simulink model "ofdm_txrx_supermimo"  (Simulink 7.1)
settings: Solver=VariableStepDiscrete  SolverMode=SingleTasking  StartTime=0.0  StopTime=simOnly_simLength  RelTol=1e-3
PostLoadFcn:
  | ofdm_rx_supermimo_init
InitFcn:
  | ofdm_rx_supermimo_init
StartFcn:
  | ofdm_rx_supermimo_init
values from neighbouring files (symbols this model uses):
  Action0_Config = 0* 31*2^18 + 0*2^24 + PHY_AUTORESPONSE_REQ_GOODHDR + PHY_AUTORESPONSE_REQ_GOODPKT + PHY_AUTORESPONSE_REQ_FLAGA    [ofdm_tx_supermimo_init.m]
  Action1_Config = 0* 62*2^18 + PHY_AUTORESPONSE_REQ_GOODHDR + PHY_AUTORESPONSE_REQ_GOODPKT    [ofdm_tx_supermimo_init.m]
  Action2_Config = 0    [ofdm_tx_supermimo_init.m]
  Action3_Config = 0    [ofdm_tx_supermimo_init.m]
  Action4_Config = 0    [ofdm_tx_supermimo_init.m]
  Action5_Config = 0    [ofdm_tx_supermimo_init.m]
  ActionID_AFTransmit = 31    [ofdm_tx_supermimo_init.m]
  ActionID_Disabled = 0    [ofdm_tx_supermimo_init.m]
  ActionID_SetFlagA = 62    [ofdm_tx_supermimo_init.m]
  ActionID_SetFlagB = 61    [ofdm_tx_supermimo_init.m]
  BER_RAM_init_size = 2048    [ofdm_tx_supermimo_init.m]
  CPLength = 16    [ofdm_tx_supermimo_init.m]
  longCorr_coef_i = [-4*real(LTS) 0]    [ofdm_rx_supermimo_init.m]
  longCorr_coef_q = [4*imag(LTS) 0]    [ofdm_rx_supermimo_init.m]
  Match0_Config = 0    [ofdm_tx_supermimo_init.m]
  Match1_Config = 0    [ofdm_tx_supermimo_init.m]
  Match2_Config = 0    [ofdm_tx_supermimo_init.m]
  Match3_Config = 0    [ofdm_tx_supermimo_init.m]
  Match4_Config = 0    [ofdm_tx_supermimo_init.m]
  Match5_Config = 0    [ofdm_tx_supermimo_init.m]
  Match6_Config = 0    [ofdm_tx_supermimo_init.m]
  Match7_Config = 0    [ofdm_tx_supermimo_init.m]
  max_CP_length = 16    [ofdm_tx_supermimo_init.m]
  max_num_baseRateSymbols = 31    [ofdm_tx_supermimo_init.m]
  max_num_subcarriers = 64    [ofdm_tx_supermimo_init.m]
  max_num_trainingSymbols = 15    [ofdm_tx_supermimo_init.m]
  max_numBytes = 16384    [ofdm_tx_supermimo_init.m]
  max_OFDM_symbols = 511    [ofdm_tx_supermimo_init.m]
  modConstellation_qam16 = .75*[1 3 -1 -3]./3    [ofdm_tx_supermimo_init.m]
  modConstellation_qam64 = 0.875*[3 1 5 7 -3 -1 -5 -7]./7    [ofdm_tx_supermimo_init.m]
  modConstellation_qpsk = [1 -1]./sqrt(2)    [ofdm_tx_supermimo_init.m]
  numBaseRateSymbols = ceil(numHeaderBytes / numBytes_BaseRateOFDMSymbol)    [ofdm_tx_supermimo_init.m]
  numSubcarriers = 64    [ofdm_tx_supermimo_init.m]
  numTrainingSymbols = 2    [ofdm_tx_supermimo_init.m]
  pilot_indicies = 7 + ( (64-21) * 2^8) + ( (64-7) * 2^16) + (21 * 2^24)    (= 356068103)    [ofdm_tx_supermimo_init.m]
  pilotValues = hex2dec('A57D') + (2^16 * hex2dec('5A82'))    [ofdm_tx_supermimo_init.m]
  pkt_crossCorr_thresh = 0.7    [ofdm_rx_supermimo_init.m]
  pkt_energy_thresh = 0    [ofdm_rx_supermimo_init.m]
  pktByteNums = numHeaderBytes +  				byteIndex_numPayloadBytes(1)*2^8 +  				byteIndex_numPayloadBytes(2)*2^16 +  				byteIndex_simpleDynModMasks * 2^24    [ofdm_rx_supermimo_init.m]
  PktDet_Delay = 58+32 +      Symbol_Timing_Offset*2^7 +      0*2^16 +      (117* 2^24)    (= 1962935642)    [ofdm_rx_supermimo_init.m]
  pktTiming_count_payloadStart = pktTiming_count_lsStart + pktTiming_ls_num*pktTiming_ls_length    [ofdm_rx_supermimo_init.m]
  preamble = preamble_scale*[shortsyms_10 longsyms_2]    [ofdm_tx_supermimo_init.m]
  preamble_ROM = preamble_I_ROM + preamble_Q_ROM*2^16    [ofdm_tx_supermimo_init.m]
  RAM_init_size = max_numBytes    (= 16384)    [ofdm_tx_supermimo_init.m]
  reg_AF_Tx_Blanking = (320+80) + ( (320+80+79)*2^16)    (= 31392144)    [ofdm_rx_supermimo_init.m]
  reg_AF_Tx_Scaling = 2944    [ofdm_rx_supermimo_init.m]
  reg_Interrupt_PktBuf_Control = reg_InterruptControl +  	1 * 2^16 +  	0 * 2^24    (= 65656)    [ofdm_rx_supermimo_init.m]
  reg_PilotCalcParams = (4 * 2^16) + (minPilotChanMag)    (= 262184.96)    [ofdm_rx_supermimo_init.m]
  regRx_chanEst_minMag = ((0.5)*2^15) + ((0.5 * 2^15)*2^16)    (= 1073758208)    [ofdm_rx_supermimo_init.m]
  regRx_coarseCalcCorrection = round((1.3e-3 - 1.2982e-3)*2^32)    [ofdm_rx_supermimo_init.m]
  regRx_pilotCalcCorrection = round(1.00115 * 2^31)    [ofdm_rx_supermimo_init.m]
  regRx_preCFOoptions = 0 * 2^0 +  	1 * 2^1 +  	0    (= 2)    [ofdm_rx_supermimo_init.m]
  rx_controlBits = 1 * 2  		 + 1 * 4  		 + 0 * 8  		 + 1 * 16 * rx_SISO_Mode  		 + 1 * 32  		 + 1 * 64  		 + 1 * 128  		 + 1 * 256  		 + 1 * 512  		 + 1 * 1024  		 + 1 * 4096  		 + 0 * 16384  		 + 0 * 32768  		 + 1 * 65536  		 + 0 * 131072  		 + 0 * 262144  		 + 1 * 524288  		 + 1 * 1048576 * tx_Alamouti_Mode  		 + 0 * 2097152           + 1 * 33554432  		 + 0 * 4194304  		 + 0 * 8388608  		 + 0 * 16777216  		 + 1 *  ...(+49 chars)    [ofdm_rx_supermimo_init.m]
  Rx_PktDet_LongCorr_Params = LongCorrCounterLoad + 0*2^8 + 0*2^16 + 255*2^24    (= 4278190325)    [ofdm_rx_supermimo_init.m]
  Rx_PktDet_LongCorrThresholds = 8000    [ofdm_rx_supermimo_init.m]
  rx_postEq_scaling = round(rxScaling*2^11) * (1 + 2^16)    [ofdm_rx_supermimo_init.m]
  RxDataScrambling_Seq = [40 198 78 63 82 173 102 245 48 111 172 115 147 230 216 93 72 65 62 2 205 242 122 90 128 83 105 97 73 10 5 252]    [ofdm_tx_supermimo_init.m]
  RxReg_FixedPktLen = 0    [ofdm_rx_supermimo_init.m]
  shortSymbol_time = shortSymbol_time(1:16).'    [ofdm_tx_supermimo_init.m]
  simOnly_numSamples = length(preamble)+( (numSubcarriers+CPLength)*(numTrainingSymbols + numBaseRateSymbols + numFullRateSymbols/2) )    [ofdm_tx_supermimo_init.m]
  train = [train train]    [ofdm_tx_supermimo_init.m]
  tx_controlBits = (antB_preambleShift * 2^4) + (2^1 * tx_Alamouti_Mode) +  	(2^0 * tx_SISO_Mode) + 4*0 + 8*1 + 0*256 + 512*0 + 1024*0 +  	0*hex2dec('1000') + 0*hex2dec('2000') + 0*hex2dec('4000') +  	1*hex2dec('8000') + 1*hex2dec('10000') + hex2dec('20000') +  	16*hex2dec('100000')    [ofdm_tx_supermimo_init.m]
  tx_delays = (50*2^0) + (0*2^8) + (30*2^12)    (= 122930)    [ofdm_tx_supermimo_init.m]
  tx_scaling = (3072) + (12288 * 2^16)    (= 805309440)    [ofdm_tx_supermimo_init.m]
  TxDataScrambling_Seq = [40 198 78 63 82 173 102 245 48 111 172 115 147 230 216 93 72 65 62 2 205 242 122 90 128 83 105 97 73 10 5 252]    [ofdm_tx_supermimo_init.m]
  txfilt_firpm = 0.95*txfilt_firpm./(max(txfilt_firpm))    [ofdm_tx_supermimo_init.m]
  txfilt_intfilt = [0 intfilt(4,4,.8)]    [ofdm_tx_supermimo_init.m]
  txReg_symbolCounts = (2^8 *numBaseRateSymbols ) + numTrainingSymbols    [ofdm_tx_supermimo_init.m]
  TxRx_FFTScaling = (3 * 2^0) +  	(2 * 2^2) +  	(1 * 2^4) +  	(1 * 2^6) +  	(1 * 2^8) +  	(0 * 2^10)    (= 347)    [ofdm_tx_supermimo_init.m]
undefined symbols (not defined in the file or in its folder): AntA, AntB, BaseRate, Down, gcbh, numBuffs, numBytes, Up, xlBlockLoadCallback, xlGetSimulinkPeriod, xlipgetpartsetting
libraries: xbsIndex_r4 x6035, simulink x4, dspsigops x4, commchan3 x2, dspsrcs4 x2, commrflib2 x2
(part 1 of 61)
settings Model: Creator=murphpo  LastModifiedBy=Administrator
settings DataIOCC: LimitDataPoints=off  SaveOutput=off  SignalLogging=off  SaveTime=off
settings OptimizationCC: InlineParams=on
settings DebuggingCC: SaveWithParameterizedLinksMsg=none

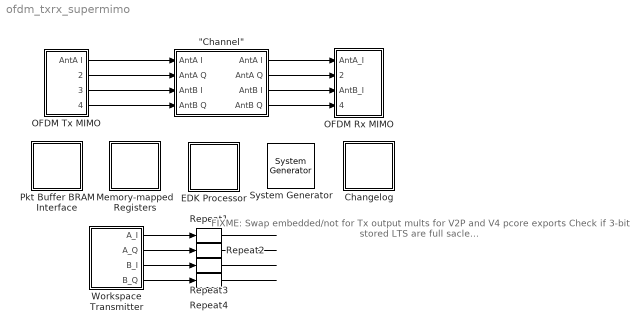
== system ofdm_txrx_supermimo ==
  note: FIXME: Swap embedded/not
      | for Tx output mults for V2P and V4
      | pcore exports
      |
      | Check if 3-bit stored LTS are full sacle...
  " System Generator" : xbsIndex_r4/ System Generator  Tag=genX  SystemSampleTime=-1  xilinxfamily=virtex2p  part=xc2vp70  speed=-6  package=ff1517  synthesis_tool=XST  clock_wrapper=Clock Enables  directory=./OFDM_txrx_v15_v50  testbench=off  simulink_period=1  sysclk_period=10  dcm_input_clock_period=100  incr_netlist=off  trim_vbits=Everywhere in SubSystem  dbl_ovrd=According to Block Masks  core_generation=According to Block Masks  run_coregen=off  deprecated_control=off  eval_field=0  block_type=sysgen
      settings: block_version=10.1.3
  "\"Channel\"" : Subsystem  mask{h=[1 0; 1 0] * 0.45 %[1 0; 0 0] * 0.5 %, freqOffsetA=0, freqOffsetB=0, awgnSNR=500, phNoise=-5000}
      mask prompts: h "2x2 Channel Matrix"; freqOffsetA "Freq Offset A (phase inc [0,1])"; freqOffsetB "Freq Offset B (phase inc [0,1])"; awgnSNR "SNR (dB)"; phNoise "Phase Noise (dBc @ 100Hz)"
      mask help: This block implements a simple static 2x2 channel. The one parameter sets the 2x2 channel matrix. h=[1 0;0 1] would be perfectly orthogonal channels. The norm of the matrix should be 1 to avoid overflow.
  Changelog : Subsystem
  EDK Processor : Subsystem  LoadFcn=xlBlockLoadCallback(gcbh);  mask(Xilinx EDK Processor Block){mode=EDK pcore generation, xmp=, MemVisToProc=<qt bgcolor="#FFFFFF"><div><img src="C:\Xilinx\10.1\DSP_Tools\common\bin\..\..\sysgen\data\images\registerplus.gif"> &lt;&lt;Rx_AF_Blanking_w&gt;&gt;<br></div><div><img src="C:\Xilinx\10.1\DSP_Tools\common\bin\..\..\sysgen\data\images\registerplus.gif"> &lt;&lt;Rx_AF_TxScaling_w&gt;&gt;<br></div><div><img src="C:\Xilinx\10.1\DSP_Tools\common\bin\..\..\sysgen\data\images\registerplus.gif"> &lt;&lt;Rx_BER_Errors&gt;&gt;<br></div><div><img src="C:\Xilinx\10.1\DSP_Tools\common\bin\..\..\sysgen\data\images\registerplus.gif"> &lt;&lt;Rx_BER_TotalBits&gt;&gt;<br></div><div><img src="C:\Xilinx\10.1\DSP_Tools\common\bin\..\..\sysgen\data\images\registerplus.gif"> &lt;&lt;Rx_ChanEst_MinMag_w&gt;&gt;<br></div><div><img src="C:\Xilinx\10.1\DSP_Tools\common\bin\..\..\sysgen\data\images\registerplus.gif"> &lt;&lt;Rx_Constellation_Scaling_w&gt;&gt;<br></div><div><img src="C:\Xilinx\10.1\DSP_Tools\common\bin\..\..\sysgen\data\images\registerplus.gif"> &lt;&lt;Rx_ControlBits_r&gt;&gt;<br></div><div><img src="C:\Xilinx\10.1\DSP_Tools\common\bin\..\..\sysgen\data\images\registerplus.gif"> &lt;&lt;Rx_ControlBits_w&gt;&gt;<br></div><div><img src="C:\Xilinx\10.1\DSP_Tools\common\bin\..\..\sysgen\data\images\registerplus.gif"> &lt;&lt;Rx_FixedPktLen_w&gt;&gt;<br></div><div><img src="C:\Xilinx\10.1\DSP_Tools\common\bin\..\..\sysgen\data\images\registerplus.gif"> &lt;&lt;Rx_Gains&gt;&gt;<br></div><div><img src="C:\Xilinx\10.1\DSP_Tools\common\bin\..\..\sysgen\data\images\registerplus.gif"> &lt;&lt;Rx_OFDM_SymbolCounts_r&gt;&gt;<br></div><div><img src="C:\Xilinx\10.1\DSP_Tools\common\bin\..\..\sysgen\data\images\registerplus.gif"> &lt;&lt;Rx_OFDM_SymbolCounts_w&gt;&gt;<br></div><div><img src="C:\Xilinx\10.1\DSP_Tools\common\bin\..\..\sysgen\data\images\registerplus.gif"> &lt;&lt;Rx_PilotCalcParams_w&gt;&gt;<br></div><div><img src="C:\Xilinx\10.1\DSP_Tools\common\bin\..\..\sysgen\data\images\registerplus.gif"> &lt;&lt;Rx_PktDet_Delay_r&gt;&gt;<br></div><div><img src="C:\Xilinx\10.1\DSP_Tools\common\bin\..\..\sysgen\data\images\registerplus.gif"> &lt;&lt;Rx_PktDet_Delay_w&gt;&gt;<br></div><div><img src="C:\Xilinx\10.1\DSP_Tools\common\bin\..\..\sysgen\data\images\registerplus.gif"> &lt;&lt;Rx_PktDet_LongCorr_Params_r&gt;&gt;<br></div><div><img src="C:\Xilinx\10.1\DSP_Tools\common\bin\..\..\sysgen\data\images\registerplus.gif"> &lt;&lt;Rx_PktDet_LongCorr_Params_w&gt;&gt;<br></div><div><img src="C:\Xilinx\10.1\DSP_Tools\common\bin\..\..\sysgen\data\images\registerplus.gif"> &lt;&lt;Rx_PktDet_LongCorr_Thresholds_w&gt;&gt;<br></div><div><img src="C:\Xilinx\10.1\DSP_Tools\common\bin\..\..\sysgen\data\images\registerplus.gif"> &lt;&lt;Rx_PreCFO_Options_w&gt;&gt;<br></div><div><img src="C:\Xilinx\10.1\DSP_Tools\common\bin\..\..\sysgen\data\images\registerplus.gif"> &lt;&lt;Rx_PreCFO_PilotCalcCorrection_w&gt;&gt;<br></div><div><img src="C:\Xilinx\10.1\DSP_Tools\common\bin\..\..\sysgen\data\images\registerplus.gif"> &lt;&lt;Rx_coarseCFO_correction_w&gt;&gt;<br></div><div><img src="C:\Xilinx\10.1\DSP_Tools\common\bin\..\..\sysgen\data\images\registerplus.gif"> &lt;&lt;Rx_coarseCFOest&gt;&gt;<br></div><div><img src="C:\Xilinx\10.1\DSP_Tools\common\bin\..\..\sysgen\data\images\registerplus.gif"> &lt;&lt;Rx_pilotCFOest&gt;&gt;<br></div><div><img src="C:\Xilinx\10.1\DSP_Tools\common\bin\..\..\sysgen\data\images\registerplus.gif"> &lt;&lt;Rx_pktByteNums_r&gt;&gt;<br></div><div><img src="C:\Xilinx\10.1\DSP_Tools\common\bin\..\..\sysgen\data\images\registerplus.gif"> &lt;&lt;Rx_pktByteNums_w&gt;&gt;<br></div><div><img src="C:\Xilinx\10.1\DSP_Tools\common\bin\..\..\sysgen\data\images\registerplus.gif"> &lt;&lt;Rx_pktDetEventCount&gt;&gt;<br></div><div><img src="C:\Xilinx\10.1\DSP_Tools\common\bin\..\..\sysgen\data\images\registerplus.gif"> &lt;&lt;Rx_pktDet_Tresholds_w&gt;&gt;<br></div><div><img src="C:\Xilinx\10.1\DSP_Tools\common\bin\..\..\sysgen\data\images\registerplus.gif"> &lt;&lt;Rx_pktDone_interruptStatus&gt;&gt;<br></div><div><img src="C:\Xilinx\10.1\DSP_Tools\common\bin\..\..\sysgen\data\images\registerplus.gif"> &lt;&lt;TxRx_AutoReply_Action0_r&gt;&gt;<br></div><div><img src="C:\Xilinx\10.1\DSP_Tools\common\bin\..\..\sysgen\data\images\registerplus.gif"> &lt;&lt;TxRx_AutoReply_Action0_w&gt;&gt;<br></div><div><img src="C:\Xilinx\10.1\DSP_Tools\common\bin\..\..\sysgen\data\images\registerplus.gif"> &lt;&lt;TxRx_AutoReply_Action1_r&gt;&gt;<br></div><div><img src="C:\Xilinx\10.1\DSP_Tools\common\bin\..\..\sysgen\data\images\registerplus.gif"> &lt;&lt;TxRx_AutoReply_Action1_w&gt;&gt;<br></div><div><img src="C:\Xilinx\10.1\DSP_Tools\common\bin\..\..\sysgen\data\images\registerplus.gif"> &lt;&lt;TxRx_AutoReply_Action2_r&gt;&gt;<br></div><div><img src="C:\Xilinx\10.1\DSP_Tools\common\bin\..\..\sysgen\data\images\registerplus.gif"> &lt;&lt;TxRx_AutoReply_Action2_w&gt;&gt;<br></div><div><img src="C:\Xilinx\10.1\DSP_Tools\common\bin\..\..\sysgen\data\images\registerplus.gif"> &lt;&lt;TxRx_AutoReply_Action3_r&gt;&gt;<br></div><div><img src="C:\Xilinx\10.1\DSP_Tools\common\bin\..\..\sysgen\data\images\registerplus.gif"> &lt;&lt;TxRx_AutoReply_Action3_w&gt;&gt;<br></div><div><img src="C:\Xilinx\10.1\DSP_Tools\common\bin\..\..\sysgen\data\images\registerplus.gif"> &lt;&lt;TxRx_AutoReply_Action4_r&gt;&gt;<br></div><div><img src="C:\Xilinx\10.1\DSP_Tools\common\bin\..\..\sysgen\data\images\registerplus.gif"> &lt;&lt;TxRx_AutoReply_Action4_w&gt;&gt;<br></div><div><img src="C:\Xilinx\10.1\DSP_Tools\common\bin\..\..\sysgen\data\images\registerplus.gif"> &lt;&lt;TxRx_AutoReply_Action5_r&gt;&gt;<br></div><div><img src="C:\Xilinx\10.1\DSP_Tools\common\bin\..\..\sysgen\data\images\registerplus.gif"> &lt;&lt;TxRx_AutoReply_Action5_w&gt;&gt;<br></div><div><img src="C:\Xilinx\10.1\DSP_Tools\common\bin\..\..\sysgen\data\images\registerplus.gif"> &lt;&lt;TxRx_AutoReply_Match0_r&gt;&gt;<br></div><div><img src="C:\Xilinx\10.1\DSP_Tools\common\bin\..\..\sysgen\data\images\registerplus.gif"> &lt;&lt;TxRx_AutoReply_Match0_w&gt;&gt;<br></div><div><img src="C:\Xilinx\10.1\DSP_Tools\common\bin\..\..\sysgen\data\images\registerplus.gif"> &lt;&lt;TxRx_AutoReply_Match1_r&gt;&gt;<br></div><div><img src="C:\Xilinx\10.1\DSP_Tools\common\bin\..\..\sysgen\data\images\registerplus.gif"> &lt;&lt;TxRx_AutoReply_Match1_w&gt;&gt;<br></div><div><img src="C:\Xilinx\10.1\DSP_Tools\common\bin\..\..\sysgen\data\images\registerplus.gif"> &lt;&lt;TxRx_AutoReply_Match2_r&gt;&gt;<br></div><div><img src="C:\Xilinx\10.1\DSP_Tools\common\bin\..\..\sysgen\data\images\registerplus.gif"> &lt;&lt;TxRx_AutoReply_Match2_w&gt;&gt;<br></div><div><img src="C:\Xilinx\10.1\DSP_Tools\common\bin\..\..\sysgen\data\images\registerplus.gif"> &lt;&lt;TxRx_AutoReply_Match3_r&gt;&gt;<br></div><div><img src="C:\Xilinx\10.1\DSP_Tools\common\bin\..\..\sysgen\data\images\registerplus.gif"> &lt;&lt;TxRx_AutoReply_Match3_w&gt;&gt;<br></div><div><img src="C:\Xilinx\10.1\DSP_Tools\common\bin\..\..\sysgen\data\images\registerplus.gif"> &lt;&lt;TxRx_AutoReply_Match4_r&gt;&gt;<br></div><div><img src="C:\Xilinx\10.1\DSP_Tools\common\bin\..\..\sysgen\data\images\registerplus.gif"> &lt;&lt;TxRx_AutoReply_Match4_w&gt;&gt;<br></div><div><img src="C:\Xilinx\10.1\DSP_Tools\common\bin\..\..\sysgen\data\images\registerplus.gif"> &lt;&lt;TxRx_AutoReply_Match5_r&gt;&gt;<br></div><div><img src="C:\Xilinx\10.1\DSP_Tools\common\bin\..\..\sysgen\data\images\registerplus.gif"> &lt;&lt;TxRx_AutoReply_Match5_w&gt;&gt;<br></div><div><img src="C:\Xilinx\10.1\DSP_Tools\common\bin\..\..\sysgen\data\images\registerplus.gif"> &lt;&lt;TxRx_AutoReply_Match6_r&gt;&gt;<br></div><div><img src="C:\Xilinx\10.1\DSP_Tools\common\bin\..\..\sysgen\data\images\registerplus.gif"> &lt;&lt;TxRx_AutoReply_Match6_w&gt;&gt;<br></div><div><img src="C:\Xilinx\10.1\DSP_Tools\common\bin\..\..\sysgen\data\images\registerplus.gif"> &lt;&lt;TxRx_AutoReply_Match7_r&gt;&gt;<br></div><div><img src="C:\Xilinx\10.1\DSP_Tools\common\bin\..\..\sysgen\data\images\registerplus.gif"> &lt;&lt;TxRx_AutoReply_Match7_w&gt;&gt;<br></div><div><img src="C:\Xilinx\10.1\DSP_Tools\common\bin\..\..\sysgen\data\images\registerplus.gif"> &lt;&lt;TxRx_FFT_Scaling_w&gt;&gt;<br></div><div><img src="C:\Xilinx\10.1\DSP_Tools\common\bin\..\..\sysgen\data\images\registerplus.gif"> &lt;&lt;TxRx_Interrupt_PktBuf_Ctrl_r&gt;&gt;<br></div><div><img src="C:\Xilinx\10.1\DSP_Tools\common\bin\..\..\sysgen\data\images\registerplus.gif"> &lt;&lt;TxRx_Interrupt_PktBuf_Ctrl_w&gt;&gt;<br></div><div><img src="C:\Xilinx\10.1\DSP_Tools\common\bin\..\..\sysgen\data\images\registerplus.gif"> &lt;&lt;TxRx_Pilots_Index_w&gt;&gt;<br></div><div><img src="C:\Xilinx\10.1\DSP_Tools\common\bin\..\..\sysgen\data\images\registerplus.gif"> &lt;&lt;TxRx_Pilots_Values_w&gt;&gt;<br></div><div><img src="C:\Xilinx\10.1\DSP_Tools\common\bin\..\..\sysgen\data\images\registerplus.gif"> &lt;&lt;Tx_ControlBits_r&gt;&gt;<br></div><div><img src="C:\Xilinx\10.1\DSP_Tools\common\bin\..\..\sysgen\data\images\registerplus.gif"> &lt;&lt;Tx_ControlBits_w&gt;&gt;<br></div><div><img src="C:\Xilinx\10.1\DSP_Tools\common\bin\..\..\sysgen\data\images\registerplus.gif"> &lt;&lt;Tx_Delays_r&gt;&gt;<br></div><div><img src="C:\Xilinx\10.1\DSP_Tools\common\bin\..\..\sysgen\data\images\registerplus.gif"> &lt;&lt;Tx_Delays_w&gt;&gt;<br></div><div><img src="C:\Xilinx\10.1\DSP_Tools\common\bin\..\..\sysgen\data\images\registerplus.gif"> &lt;&lt;Tx_OFDM_SymCounts_r&gt;&gt;<br></div><div><img src="C:\Xilinx\10.1\DSP_Tools\common\bin\..\..\sysgen\data\images\registerplus.gif"> &lt;&lt;Tx_OFDM_SymCounts_w&gt;&gt;<br></div><div><img src="C:\Xilinx\10.1\DSP_Tools\common\bin\..\..\sysgen\data\images\registerplus.gif"> &lt;&lt;Tx_PktRunning&gt;&gt;<br></div><div><img src="C:\Xilinx\10.1\DSP_Tools\common\bin\..\..\sysgen\data\images\registerplus.gif"> &lt;&lt;Tx_Scaling_w&gt;&gt;<br></div><div><img src="C:\Xilinx\10.1\DSP_Tools\common\bin\..\..\sysgen\data\images\registerplus.gif"> &lt;&lt;Tx_Start_Reset_Control_r&gt;&gt;<br></div><div><img src="C:\Xilinx\10.1\DSP_Tools\common\bin\..\..\sysgen\data\images\registerplus.gif"> &lt;&lt;Tx_Start_Reset_Control_w&gt;&gt;<br></div><div><img src="C:\Xilinx\10.1\DSP_Tools\common\bin\..\..\sysgen\data\images\registerplus.gif"> &lt;&lt;midPacketRSSI&gt;&gt;<br></div><div><img src="C:\Xilinx\10.1\DSP_Tools\common\bin\..\..\sysgen\data\images\registerplus.gif"> &lt;&lt;pktDet_controlBits_r&gt;&gt;<br></div><div><img src="C:\Xilinx\10.1\DSP_Tools\common\bin\..\..\sysgen\data\images\registerplus.gif"> &lt;&lt;pktDet_controlBits_w&gt;&gt;<br></div><div><img src="C:\Xilinx\10.1\DSP_Tools\common\bin\..\..\sysgen\data\images\registerplus.gif"> &lt;&lt;pktDet_durations_r&gt;&gt;<br></div><div><img src="C:\Xilinx\10.1\DSP_Tools\common\bin\..\..\sysgen\data\images\registerplus.gif"> &lt;&lt;pktDet_durations_w&gt;&gt;<br></div><div><img src="C:\Xilinx\10.1\DSP_Tools\common\bin\..\..\sysgen\data\images\registerplus.gif"> &lt;&lt;pktDet_status&gt;&gt;<br></div><div><img src="C:\Xilinx\10.1\DSP_Tools\common\bin\..\..\sysgen\data\images\registerplus.gif"> &lt;&lt;pktDet_thresholds_r&gt;&gt;<br></div><div><img src="C:\Xilinx\10.1\DSP_Tools\common\bin\..\..\sysgen\data\images\registerplus.gif"> &lt;&lt;pktDet_thresholds_w&gt;&gt;<br></div><div><img src="C:\Xilinx\10.1\DSP_Tools\common\bin\..\..\sysgen\data\images\memoryplus.gif"> &lt;&lt;ChannelEstimates&gt;&gt;<br></div><div><img src="C:\Xilinx\10.1\DSP_Tools\common\bin\..\..\sysgen\data\images\memoryplus.gif"> &lt;&lt;EVM_perSC&gt;&gt;<br></div><div><img src="C:\Xilinx\10.1\DSP_Tools\common\bin\..\..\sysgen\data\images\memoryplus.gif"> &lt;&lt;EVM_perSym&gt;&gt;<br></div><div><img src="C:\Xilinx\10.1\DSP_Tools\common\bin\..\..\sysgen\data\images\memoryplus.gif"> &lt;&lt;PktBufFreqOffsets&gt;&gt;<br></div><div><img src="C:\Xilinx\10.1\DSP_Tools\common\bin\..\..\sysgen\data\images\memoryplus.gif"> &lt;&lt;RxModulation&gt;&gt;<br></div><div><img src="C:\Xilinx\10.1\DSP_Tools\common\bin\..\..\sysgen\data\images\memoryplus.gif"> &lt;&lt;TxHeaderTranslate&gt;&gt;<br></div><div><img src="C:\Xilinx\10.1\DSP_Tools\common\bin\..\..\sysgen\data\images\memoryplus.gif"> &lt;&lt;TxModulation&gt;&gt;<br></div></qt>, AvailableMemories=<empty>, portInterfaceTable={'exposed'=>[],'portdir'=>[],'portname'=>[],'shortname'=>[]}, bus_type_sgadvanced=, bus_type=PLB, baseaddr=0x80000000, baseaddr_lock_sgadvanced=, baseaddr_lock=off, dual_clock_sgadvanced=, dual_clock=off, reg_readback_sgadvanced=, reg_readback=off, ucf_file=, inheritDeviceType_sgadvanced=, inheritDeviceType=off, clock_name=plb, internalPortList={}, resetPolarity=0, memxtable=|, procinfo={'xmliface'=>'Xilinx//microblaze//iface.xml'}, memmapdirty=off, blockname=, xpsintstyle=default, has_advanced_control=0, sggui_pos=20,20,383,441, block_type=edkprocessor, block_version=2.5, sg_icon_stat=47,45,-1,-1,white,blue,0,07734,right, sg_mask_display=|, sg_list_contents={'table'=>{'AvailableMemories'=>'popup(<empty>)','userSelections'=>{'AvailableMemories'=>'<empty>'}}}, sg_blockgui_xml=}
      mask memxtable=|
      | {'mlist'=>['ofdm_txrx_supermimo/Memory-mapped
      | Registers/Rx Registers/Write-Only Register4/From Register','ofdm_txrx_supermimo/Memory-mapped
      | Registers/Rx Registers/Write-Only Register6/From Register','ofdm_txrx_supermimo/Memory-mapped
      | Registers/Rx Registers/Read Only Register1/To Register','ofdm_txrx_supermimo/Memory-mapped
      | Registers/Rx Registers/Read Only Register2/To Register','ofdm_txrx_supermimo/Memory-mapped
      | Registers/Rx Registers/Write-Only Register8/From Register','ofdm_txrx_supermimo/Memory-mapped
      | Registers/Rx Registers/Write-Only Register1/From Register','ofdm_txrx_supermimo/Memory-mapped
      | Registers/Rx Registers/Read-Write Register/To Register','ofdm_txrx_supermimo/Memory-mapped
      | Registers/Rx Registers/Read-Write Register/From Register','ofdm_txrx_supermimo/Memory-mapped
      | Registers/Rx Registers/Write-Only Register10/From Register','ofdm_txrx_supermimo/Memory-mapped
      | Registers/Rx Registers/Read Only Register/To Register','ofdm_txrx_supermimo/Memory-mapped
      | Registers/TxRx Registers/Read-Write Register4/To Register','ofdm_txrx_supermimo/Memory-mapped
      | Registers/TxRx Registers/Read-Write Register4/From Register','ofdm_txrx_supermimo/Memory-mapped
      | Registers/Rx Registers/Write-Only Register5/From Register','ofdm_txrx_supermimo/Memory-mapped
      | Registers/Rx Registers/Read-Write Register3/To Register','ofdm_txrx_supermimo/Memory-mapped
      | Registers/Rx Registers/Read-Write Register3/From Register','ofdm_txrx_supermimo/Memory-mapped
      | Registers/Rx Registers/Read-Write Register1/To Register','ofdm_txrx_supermimo/Memory-mapped
      | Registers/Rx Registers/Read-Write Register1/From Register','ofdm_txrx_supermimo/Memory-mapped
      | Registers/Rx Registers/Write-Only Register9/From Register','ofdm_txrx_supermimo/Memory-mapped
      | Registers/Rx Registers/Write-Only Register7/From Register','ofdm_txrx_supermimo/Memory-mapped
      | Registers/Rx Registers/Write-Only Register2/From Register','ofdm_txrx_supermimo/Memory-mapped
      | Registers/Rx Registers/Write-Only Register3/From Register','ofdm_txrx_supermimo/Memory-mapped
      | Registers/Rx Registers/Read Only Register5/To Register','ofdm_txrx_supermimo/Memory-mapped
      | Registers/Rx Registers/Read Only Register4/To Register','ofdm_txrx_supermimo/Memory-mapped
      | Registers/Rx Registers/Read-Write Register2/To Register','ofdm_txrx_supermimo/Memory-mapped
      | Registers/Rx Registers/Read-Write Register2/From Register','ofdm_txrx_supermimo/Memory-mapped
      | Registers/Rx Registers/Read Only Register6/To Register','ofdm_txrx_supermimo/Memory-mapped
      | Registers/Rx Registers/Write-Only Register/From Register','ofdm_txrx_supermimo/Memory-mapped
      | Registers/Rx Registers/Read Only Register3/To Register','ofdm_txrx_supermimo/Memory-mapped
      | Registers/AutoReply Registers/Read-Write Register7/To Register','ofdm_txrx_supermimo/Memory-mapped
      | Registers/AutoReply Registers/Read-Write Register7/From Register','ofdm_txrx_supermimo/Memory-mapped
      | Registers/AutoReply Registers/Read-Write Register8/To Register','ofdm_txrx_supermimo/Memory-mapped
      | Registers/AutoReply Registers/Read-Write Register8/From Register','ofdm_txrx_supermimo/Memory-mapped
      | Registers/AutoReply Registers/Read-Write Register9/To Register','ofdm_txrx_supermimo/Memory-mapped
      | Registers/AutoReply Registers/Read-Write Register9/From Register','ofdm_txrx_supermimo/Memory-mapped
      | Registers/AutoReply Registers/Read-Write Register10/To Register','ofdm_txrx_supermimo/Memory-mapped
      | Registers/AutoReply Registers/Read-Write Register10/From Register','ofdm_txrx_supermimo/Memory-mapped
      | Registers/AutoReply Registers/Read-Write Register12/To Register','ofdm_txrx_supermimo/Memory-mapped
      | Registers/AutoReply Registers/Read-Write Register12/From Register','ofdm_txrx_supermimo/Memory-mapped
      | Registers/AutoReply Registers/Read-Write Register11/To Register','ofdm_txrx_supermimo/Memory-mapped
      | Registers/AutoReply Registers/Read-Write Register11/From Register','ofdm_txrx_supermimo/Memory-mapped
      | Registers/AutoReply Registers/Read-Write Register5/To Register','ofdm_txrx_supermimo/Memory-mapped
      | Registers/AutoReply Registers/Read-Write Register5/From Register','ofdm_txrx_supermimo/Memory-mapped
      | Registers/AutoReply Registers/Read-Write Register1/To Register','ofdm_txrx_supermimo/Memory-mapped
      | Registers/AutoReply Registers/Read-Write Register1/From Register','ofdm_txrx_supermimo/Memory-mapped
      | Registers/AutoReply Registers/Read-Write Register2/To Register','ofdm_txrx_supermimo/Memory-mapped
      | Registers/AutoReply Registers/Read-Write Register2/From Register','ofdm_txrx_supermimo/Memory-mapped
      | Registers/AutoReply Registers/Read-Write Register6/To Register','ofdm_txrx_supermimo/Memory-mapped
      | Registers/AutoReply Registers/Read-Write Register6/From Register','ofdm_txrx_supermimo/Memory-mapped
      | Registers/AutoReply Registers/Read-Write Register3/To Register','ofdm_txrx_supermimo/Memory-mapped
      | Registers/AutoReply Registers/Read-Write Register3/From Register','ofdm_txrx_supermimo/Memory-mapped
      | Registers/AutoReply Registers/Read-Write Register4/To Register','ofdm_txrx_supermimo/Memory-mapped
      | Registers/AutoReply Registers/Read-Write Register4/From Register','ofdm_txrx_supermimo/Memory-mapped
      | Registers/AutoReply Registers/Read-Write Register13/To Register','ofdm_txrx_supermimo/Memory-mapped
      | Registers/AutoReply Registers/Read-Write Register13/From Register','ofdm_txrx_supermimo/Memory-mapped
      | Registers/AutoReply Registers/Read-Write Register14/To Register','ofdm_txrx_supermimo/Memory-mapped
      | Registers/AutoReply Registers/Read-Write Register14/From Register','ofdm_txrx_supermimo/Memory-mapped
      | Registers/TxRx Registers/Write-Only Register/From Register','ofdm_txrx_supermimo/Memory-mapped
      | Registers/TxRx Registers/Read-Write Register/To Register','ofdm_txrx_supermimo/Memory-mapped
      | Registers/TxRx Registers/Read-Write Register/From Register','ofdm_txrx_supermimo/Memory-mapped
      | Registers/TxRx Registers/Write-Only Register2/From Register','ofdm_txrx_supermimo/Memory-mapped
      | Registers/TxRx Registers/Write-Only Register4/From Register','ofdm_txrx_supermimo/Memory-mapped
      | Registers/Tx Registers/Read-Write Register1/To Register','ofdm_txrx_supermimo/Memory-mapped
      | Registers/Tx Registers/Read-Write Register1/From Register','ofdm_txrx_supermimo/Memory-mapped
      | Registers/Tx Registers/Read-Write Register2/To Register','ofdm_txrx_supermimo/Memory-mapped
      | Registers/Tx Registers/Read-Write Register2/From Register','ofdm_txrx_supermimo/Memory-mapped
      | Registers/TxRx Registers/Read-Write Register2/To Register','ofdm_txrx_supermimo/Memory-mapped
      | Registers/TxRx Registers/Read-Write Register2/From Register','ofdm_txrx_supermimo/Memory-mapped
      | Registers/Tx Registers/Read Only Register/To Register','ofdm_txrx_supermimo/Memory-mapped
      | Registers/Tx Registers/Write-Only Register1/From Register','ofdm_txrx_supermimo/Memory-mapped
      | Registers/Tx Registers/Read-Write Register/To Register','ofdm_txrx_supermimo/Memory-mapped
      | Registers/Tx Registers/Read-Write Register/From Register','ofdm_txrx_supermimo/OFDM Rx MIMO/Packet_Detection/RSSI-based PktDet/Mid-Packet
      | RSSI Register/To Register2','ofdm_txrx_supermimo/Memory-mapped
      | Registers/Rx Registers/Pkt Detector Registers/Read-Write Register1/To Register','ofdm_txrx_supermimo/Memory-mapped
      | Registers/Rx Registers/Pkt Detector Registers/Read-Write Register1/From Register','ofdm_txrx_supermimo/Memory-mapped
      | Registers/Rx Registers/Pkt Detector Registers/Read-Write Register2/To Register','ofdm_txrx_supermimo/Memory-mapped
      | Registers/Rx Registers/Pkt Detector Registers/Read-Write Register2/From Register','ofdm_txrx_supermimo/Memory-mapped
      | Registers/Rx Registers/Pkt Detector Registers/To Register','ofdm_txrx_supermimo/Memory-mapped
      | Registers/Rx Registers/Pkt Detector Registers/Read-Write Register5/To Register','ofdm_txrx_supermimo/Memory-mapped
      | Registers/Rx Registers/Pkt Detector Registers/Read-Write Register5/From Register','ofdm_txrx_supermimo/OFDM Rx MIMO/FFT & Chan Est/Channel Estimation
      | & Pilot Processing/Chan Est Buffer
      | & Mag Calc/Shared Memory','ofdm_txrx_supermimo/OFDM Rx MIMO/Equalizer & Packetizer/Packet_Constructor/EVM Calc/EVM per Subcarrier/Shared Memory','ofdm_txrx_supermimo/OFDM Rx MIMO/Equalizer & Packetizer/Packet_Constructor/EVM Calc/EVM per Symbol/Shared Memory','ofdm_txrx_supermimo/OFDM Rx MIMO/Coarse Freq Correction/PreSpin CFO Sel/Shared Memory','ofdm_txrx_supermimo/OFDM Rx MIMO/Equalizer & Packetizer/Packet_Constructor/Modulation RAM/Modulation Masks/Shared Memory','ofdm_txrx_supermimo/OFDM Tx MIMO/Training_Data/FlexibleMod/PktBuffer_CRC1/Packet Buffer/AutoTx
      | Header Translation/Shared Memory','ofdm_txrx_supermimo/OFDM Tx MIMO/Training_Data/FlexibleMod/control/Tx Modulation/Shared Memory for
      | Modulation Masks/Shared Memory'],'mlname'=>['\'Rx_AF_Blanking_w\'','\'Rx_AF_TxScaling_w\'','\'Rx_BER_Errors\'','\'Rx_BER_TotalBits\'','\'Rx_ChanEst_MinMag_w\'','\'Rx_Constellation_Scaling_w\'','\'Rx_ControlBits_r\'','\'Rx_ControlBits_w\'','\'Rx_FixedPktLen_w\'','\'Rx_Gains\'','\'Rx_OFDM_SymbolCounts_r\'','\'Rx_OFDM_SymbolCounts_w\'','\'Rx_PilotCalcParams_w\'','\'Rx_PktDet_Delay_r\'','\'Rx_PktDet_Delay_w\'','\'Rx_PktDet_LongCorr_Params_r\'','\'Rx_PktDet_LongCorr_Params_w\'','\'Rx_PktDet_LongCorr_Thresholds_w\'','\'Rx_PreCFO_Options_w\'','\'Rx_PreCFO_PilotCalcCorrection_w\'','\'Rx_coarseCFO_correction_w\'','\'Rx_coarseCFOest\'','\'Rx_pilotCFOest\'','\'Rx_pktByteNums_r\'','\'Rx_pktByteNums_w\'','\'Rx_pktDetEventCount\'','\'Rx_pktDet_Tresholds_w\'','\'Rx_pktDone_interruptStatus\'','\'TxRx_AutoReply_Action0_r\'','\'TxRx_AutoReply_Action0_w\'','\'TxRx_AutoReply_Action1_r\'','\'TxRx_AutoReply_Action1_w\'','\'TxRx_AutoReply_Action2_r\'','\'TxRx_AutoReply_Action2_w\'','\'TxRx_AutoReply_Action3_r\'','\'TxRx_AutoReply_Action3_w\'','\'TxRx_AutoReply_Action4_r\'','\'TxRx_AutoReply_Action4_w\'','\'TxRx_AutoReply_Action5_r\'','\'TxRx_AutoReply_Action5_w\'','\'TxRx_AutoReply_Match0_r\'','\'TxRx_AutoReply_Match0_w\'','\'TxRx_AutoReply_Match1_r\'','\'TxRx_AutoReply_Match1_w\'','\'TxRx_AutoReply_Match2_r\'','\'TxRx_AutoReply_Match2_w\'','\'TxRx_AutoReply_Match3_r\'','\'TxRx_AutoReply_Match3_w\'','\'TxRx_AutoReply_Match4_r\'','\'TxRx_AutoReply_Match4_w\'','\'TxRx_AutoReply_Match5_r\'','\'TxRx_AutoReply_Match5_w\'','\'TxRx_AutoReply_Match6_r\'','\'TxRx_AutoReply_Match6_w\'','\'TxRx_AutoReply_Match7_r\'','\'TxRx_AutoReply_Match7_w\'','\'TxRx_FFT_Scaling_w\'','\'TxRx_Interrupt_PktBuf_Ctrl_r\'','\'TxRx_Interrupt_PktBuf_Ctrl_w\'','\'TxRx_Pilots_Index_w\'','\'TxRx_Pilots_Values_w\'','\'Tx_ControlBits_r\'','\'Tx_ControlBits_w\'','\'Tx_Delays_r\'','\'Tx_Delays_w\'','\'Tx_OFDM_SymCounts_r\'','\'Tx_OFDM_SymCounts_w\'','\'Tx_PktRunning\'','\'Tx_Scaling_w\'','\'Tx_Start_Reset_Control_r\'','\'Tx_Start_Reset_Control_w\'','\'midPacketRSSI\'','\'pktDet_controlBits_r\'','\'pktDet_controlBits_w\'','\'pktDet_durations_r\'','\'pktDet_durations_w\'','\'pktDet_status\'','\'pktDet_thresholds_r\'','\'pktDet_thresholds_w\'','\'ChannelEstimates\'','\'EVM_perSC\'','\'EVM_perSym\'','\'PktBufFreqOffsets\'','\'RxModulation\'','\'TxHeaderTranslate\'','\'TxModulation\''],'mlstate'=>[0.00000000000000000,0.00000000000000000,0.00000000000000000,0.00000000000000000,0.00000000000000000,0.00000000000000000,0.00000000000000000,0.00000000000000000,0.00000000000000000,0.00000000000000000,0.00000000000000000,0.00000000000000000,0.00000000000000000,0.00000000000000000,0.00000000000000000,0.00000000000000000,0.00000000000000000,0.00000000000000000,0.00000000000000000,0.00000000000000000,0.00000000000000000,0.00000000000000000,0.00000000000000000,0.00000000000000000,0.00000000000000000,0.00000000000000000,0.00000000000000000,0.00000000000000000,0.00000000000000000,0.00000000000000000,0.00000000000000000,0.00000000000000000,0.00000000000000000,0.00000000000000000,0.00000000000000000,0.00000000000000000,0.00000000000000000,0.00000000000000000,0.00000000000000000,0.00000000000000000,0.00000000000000000,0.00000000000000000,0.00000000000000000,0.00000000000000000,0.00000000000000000,0.00000000000000000,0.00000000000000000,0.00000000000000000,0.00000000000000000,0.00000000000000000,0.00000000000000000,0.00000000000000000,0.00000000000000000,0.00000000000000000,0.00000000000000000,0.00000000000000000,0.00000000000000000,0.00000000000000000,0.00000000000000000,0.00000000000000000,0.00000000000000000,0.00000000000000000,0.00000000000000000,0.00000000000000000,0.00000000000000000,0.00000000000000000,0.00000000000000000,0.00000000000000000,0.00000000000000000,0.00000000000000000,0.00000000000000000,0.00000000000000000,0.00000000000000000,0.00000000000000000,0.00000000000000000,0.00000000000000000,0.00000000000000000,0.00000000000000000,0.00000000000000000,0.00000000000000000,0.00000000000000000,0.00000000000000000,0.00000000000000000,0.00000000000000000,0.00000000000000000,0.00000000000000000]}
      mask sg_mask_display=|
      | fprintf('','COMMENT: begin icon graphics');
      | patch([0 47 47 0 ],[0 0 45 45 ],[0.77 0.82 0.91]);
      | patch([11 4 15 4 11 23 26 29 42 32 22 15 26 15 22 32 42 29 26 23 11 ],[5 12 23 34 41 41 38 41 41 31 41 34 23 12 5 15 5 5 8 5 5 ],[0.98 0.96 0.92]);
      | plot([0 47 47 0 0 ],[0 0 45 45 0 ]);
      | fprintf('','COMMENT: end icon graphics');
      | fprintf('','COMMENT: begin icon text');
      | fprintf('','COMMENT: end icon text');
      mask prompts: mode "Configure Processor for"; xmp "EDK Project"; MemVisToProc ""; AvailableMemories "Available Memories"; portInterfaceTable ""; bus_type_sgadvanced ""; bus_type "Bus Type"; baseaddr "Base Address"; baseaddr_lock_sgadvanced ""; baseaddr_lock "Lock"; dual_clock_sgadvanced ""; dual_clock "Dual Clocks"; reg_readback_sgadvanced ""; reg_readback "Register Read-Back"; ucf_file "Constraint file"; inheritDeviceType_sgadvanced ""; inheritDeviceType "Inherit Device Type"; clock_name ""; internalPortList ""; resetPolarity ""; memxtable ""; procinfo ""; memmapdirty ""; blockname ""; xpsintstyle ""; has_advanced_control ""; sggui_pos ""; block_type ""; block_version ""; sg_icon_stat ""; sg_mask_display ""; sg_list_contents ""; sg_blockgui_xml ""
      mask help: Xilinx EDK Processor
      mask init:
      | try
      |   tmp_gcb = gcb;
      |   tmp_gcbh = gcbh;
      |   if (strcmp('SysGenIndex',get_param(bdroot(tmp_gcbh),'tag')) && ~isempty(regexp(bdroot(tmp_gcb), '^xbs', 'once')))
      |     return;
      |   end;
      |   xlMungeMaskParams;
      | 
      | block_type='edkprocessor';
      |   serialized_declarations = '{,''block_type''=>''String''}';
      |   xledkprocessor_init();
      |   ptable_ = xlblockprep(get_param(tmp_gcb, 'MaskWSVariables'));
      | catch
      |   global dbgsysgen;
      |   if(~isempty(dbgsysgen) && dbgsysgen)
      |     e = regexprep(lasterr, '\n', '\nError: ');
      |     disp(['Error: While running MaskInit code on block ' tmp_gcb ': ' e]);
      |     error(e);
      |   end
      | end
      settings: CopyFcn=xlProcBlockCopyCallback(gcbh);xlBlockMoveCallback(gcbh);  DeleteFcn=xlDestroyGui(gcbh);  ModelCloseFcn=xlDestroyGui(gcbh);  PreSaveFcn=xlBlockPreSaveCallback(gcbh);  PostSaveFcn=xlBlockPostSaveCallback(gcbh);  DestroyFcn=xlDestroyGui(gcbh);  OpenFcn=bh=gcbh;xlProcBlockCallbacks('populatesharedmemorylistbox',bh);xlOpenGui(bh, 'edkprocessor_gui.xml', @xlProcBlockEnablement, @xlProcBlockAction)  CloseFcn=xlDestroyGui(gcbh);  MoveFcn=xlBlockMoveCallback(gcbh);
      mask Help: eval('');xlDoc('-book','sysgen','-topic','EDK_Processor');
      mask StyleString: popup(EDK pcore generation|HDL netlisting),edit,edit,popup(<empty>),edit,edit,popup(PLB|FSL),edit,edit,checkbox,edit,checkbox,edit,checkbox,edit,edit,checkbox,edit,edit,edit,edit,edit,edit,edit,edit,edit,edit,edit,edit,edit,edit,edit,edit
      mask Display:
      | fprintf('','COMMENT: begin icon graphics');
      | patch([0 47 47 0 ],[0 0 45 45 ],[0.77 0.82 0.91]);
      | patch([11 4 15 4 11 23 26 29 42 32 22 15 26 15 22 32 42 29 26 23 11 ],[5 12 23 34 41 41 38 41 41 31 41 34 23 12 5 15 5 5 8 5 5 ],[0.98 0.96 0.92]);
      | plot([0 47 47 0 0 ],[0 0 45 45 0 ]);
      | fprintf('','COMMENT: end icon graphics');
      | fprintf('','COMMENT: begin icon text');
      | disp('');
      | 
      | fprintf('','COMMENT: end icon text');
  Memory-mapped Registers : Subsystem
  OFDM Rx MIMO : Subsystem
  OFDM Tx MIMO : Subsystem
  Pkt Buffer BRAM Interface : Subsystem
  Repeat{1..4} (4 alike) : dspsigops/Repeat  N=4  ic=0  mode=Maintain input frame size
  Workspace Transmitter : Subsystem
  wires:
  "\"Channel\""/1(" AntA I") -> OFDM Rx MIMO/1(AntA_I)
  "\"Channel\""/2(" AntA Q") -> OFDM Rx MIMO/2(AntA_Q)
  "\"Channel\""/3(" AntB I") -> OFDM Rx MIMO/3(AntB_I)
  "\"Channel\""/4(" AntB Q") -> OFDM Rx MIMO/4(AntB_Q)
  OFDM Tx MIMO/1(AntA I) -> "\"Channel\""/1(AntA I)
  OFDM Tx MIMO/2(AntA Q) -> "\"Channel\""/2(AntA Q)
  OFDM Tx MIMO/3(AntB I) -> "\"Channel\""/3(AntB I)
  OFDM Tx MIMO/4(AntB Q) -> "\"Channel\""/4(AntB Q)
  Workspace Transmitter/2(A_Q) -> Repeat2/1
  Workspace Transmitter/3(B_I) -> Repeat3/1
  Workspace Transmitter/4(B_Q) -> Repeat4/1
  Repeat2/1 -> ?
  Repeat3/1 -> ?
  Repeat4/1 -> ?
  Workspace Transmitter/1(A_I) -> Repeat1/1
  Repeat1/1 -> ?

== system ofdm_txrx_supermimo/"Channel" ==
  AntA I : Inport  OutDataType=sfix(16)  OutScaling=2^0
  AntA Q : Inport  Port=2  OutDataType=sfix(16)  OutScaling=2^0
  AntB I : Inport  Port=3  OutDataType=sfix(16)  OutScaling=2^0
  AntB Q : Inport  Port=4  OutDataType=sfix(16)  OutScaling=2^0
  AWGN Channel : commchan3/AWGN Channel  SystemSampleTime=-1  seed=rand  noiseMode="Signal to noise ratio  (SNR)"  EbNodB=10  EsNodB=10  SNRdB=awgnSNR  bitsPerSym=1  Ps=1  Tsym=1  variance=1
  AWGN Channel1 : commchan3/AWGN Channel  SystemSampleTime=-1  seed=rand  noiseMode="Signal to noise ratio  (SNR)"  EbNodB=10  EsNodB=10  SNRdB=awgnSNR  bitsPerSym=1  Ps=1  Tsym=1  variance=1
  Add : Sum  Inputs=+-  OutDataType=sfix(16)  OutScaling=2^-10
  Add1 : Sum  Inputs=+-  OutDataType=sfix(16)  OutScaling=2^-10
  Complex to Magnitude-Angle{,1..3} (4 alike) : ComplexToMagnitudeAngle
  Complex to Real-Imag1 : ComplexToRealImag
  Complex to Real-Imag2 : ComplexToRealImag
  Delays : Subsystem
  Freq Offset : Subsystem
  Gain : Gain  ParameterDataType=sfix(16)  ParameterScaling=2^0  OutDataType=sfix(16)  OutScaling=2^0
  Gain1 : Gain  ParameterDataType=sfix(16)  ParameterScaling=2^0  OutDataType=sfix(16)  OutScaling=2^0
  Integer Delay : simulink/Discrete/Integer Delay  vinit=0.0  samptime=-1  NumDelays=4
  Integer Delay1 : simulink/Discrete/Integer Delay  vinit=0.0  samptime=-1  NumDelays=4
  "MIMO \"Channel\"" : Subsystem
  Phase Noise : commrflib2/Phase Noise  SystemSampleTime=-1  PhNs=phNoise  Fo=100  seed=rand
      settings: AttributesFormatString=\n
  Phase Noise1 : commrflib2/Phase Noise  SystemSampleTime=-1  PhNs=phNoise  Fo=100  seed=rand
      settings: AttributesFormatString=\n
  Real-Imag to Complex : RealImagToComplex
  Real-Imag to Complex1 : RealImagToComplex
  Zero-Order Hold{,1..3} (4 alike) : ZeroOrderHold
  " AntA I" : Outport  OutDataType=sfix(16)  OutScaling=2^0
  " AntA Q" : Outport  Port=2  OutDataType=sfix(16)  OutScaling=2^0
  " AntB I" : Outport  Port=3  OutDataType=sfix(16)  OutScaling=2^0
  " AntB Q" : Outport  Port=4  OutDataType=sfix(16)  OutScaling=2^0
  wires:
  AntA I/1 -> Zero-Order Hold/1
  AntA Q/1 -> Zero-Order Hold1/1
  AntB I/1 -> Zero-Order Hold2/1
  AntB Q/1 -> Zero-Order Hold3/1
  Complex to Real-Imag1/1 -> " AntA I"/1
  Complex to Real-Imag1/2 -> " AntA Q"/1
  Complex to Real-Imag2/1 -> " AntB I"/1
  Complex to Real-Imag2/2 -> " AntB Q"/1
  Gain/1 -> Integer Delay/1
  AWGN Channel/1 -> Complex to Real-Imag1/1
  Gain1/1 -> Integer Delay1/1
  AWGN Channel1/1 -> Complex to Real-Imag2/1
  Real-Imag to Complex/1 -> Delays/1(In1)
  Real-Imag to Complex1/1 -> Delays/2(In2)
  Phase Noise/1 -> AWGN Channel/1, Complex to Magnitude-Angle1/1
  Phase Noise1/1 -> AWGN Channel1/1, Complex to Magnitude-Angle3/1
  Integer Delay1/1 -> Phase Noise1/1, Complex to Magnitude-Angle2/1
  Integer Delay/1 -> Phase Noise/1, Complex to Magnitude-Angle/1
  Zero-Order Hold1/1 -> Real-Imag to Complex/2
  Zero-Order Hold2/1 -> Real-Imag to Complex1/1
  Zero-Order Hold3/1 -> Real-Imag to Complex1/2
  Zero-Order Hold/1 -> Real-Imag to Complex/1
  Complex to Magnitude-Angle/2 -> Add/1
  Complex to Magnitude-Angle1/2 -> Add/2
  Complex to Magnitude-Angle2/2 -> Add1/1
  Complex to Magnitude-Angle3/2 -> Add1/2
  Delays/1(Out1) -> Freq Offset/1(In1)
  Delays/2(Out2) -> Freq Offset/2(In2)
  "MIMO \"Channel\""/1(Out1) -> Gain/1
  "MIMO \"Channel\""/2(Out2) -> Gain1/1
  Freq Offset/1(Out1) -> "MIMO \"Channel\""/1(In1)
  Freq Offset/2(Out2) -> "MIMO \"Channel\""/2(In2)

== system ofdm_txrx_supermimo/Changelog ==
  note: Export 3.00.v:
      | -Fixed EVM-per-sc logic to reset at start of each Rx packet
  note: Export 3.00.w:
      | -Fixed autoResponder timing (new CRC blocks were 2 cycles slower declaring good/bad payload)
  note: Export 3.00.x:
      | -Added extra delay bits for autoTx and reTx counters
      | -Increased int. bits for per-SC EVM accumulation
      | -Added external pktDet port
      | -Rearragned TxControlBits register bits
  note: Export 3.00.y:
      | -Added additional TxRunning outputs with programmable masks/delays (for faking pktDetecton)
      | -Rearranged TxControlBits register and added TxDelays register
  note: Export 3.00.z:
      | -Added logic to allow coarse CFO to be triggered only by external pktDet (so long correlation can be bypassed entirely)
      | -Added channel estimate magnitude checking to optionally ignore HAA or HBA when they're too small
      | -Added logic to optionally mask part of the AF transmission (to blank the now-noisy unused training symbol)
      | -Changed AF scaling so all AF samples are scaled by TxPayload scaling (only re-generated preamble gets scaled
      |     by TxPreamble scaling)
  note: Export 3.01.a:
      | -Fixed timing bug that blocked autoResponder flags
  note: Export 3.01.b:
      | -Added selectable filters for TxA I/Q
  note: Export 3.01.c:
      | -Added precision to long correlator
  note: Export 3.01.d:
      | -Added registers to mask input I/Q until AGC is done
  note: Export 3.01.e:
      | -Added extra longCorr threshold check
  note: Export 3.01.f:
      | -Removed dualThreshold logic (didn't help)
      | -Rebuilt preamble generator for realy cyclic shift of STS/LTS for antB (removed masking too)
      | -Added regs to fix length of Rx pkt (for testing "true" AF)
      | -Removed unused RxControlBits (reqShortCorr, dynPktLenEn)
      | -Added windowing for LongCorr
      | -Added optional conjugation of antB preamble
      | -Added conjugate-correlation output from LongCorr
  note: Export 3.01.g:
      | -Redesigned long corr to use 1-bit inputs, 3-bit stored coefficients (avoids input amplitude dependence)
  note: Export 3.01.h:
      | -Added optional cyclic shift to Tx chain (shift happens before CP insertion, for training and data)
      | -Removed conjugation for preamble B
      | -Delayed preamble/payload scaling by 2 more (was switching to higher payload scaling too early)
      | -This export is OFDM refdes v15.0 V2P public
  note: Export 3.01.k:
      | -Replaced downsamples-with-enables in phase tracker with DFF->downsample (workaround for Sysgen Virtex-4 export bug)
      | -Made 10 multipliers non-embedded (too many mults for DSP48's in V4FX100)
      | -This export is OFDM refdes v15.0 V4 public
  note: Export 3.01.l:
      | -Reverted multiplier settings for further development on V2P
      | -This version hasn't actually been integrated in an XPS project (just a milestone post-v15 release)
  Through PHY 3.00i : Subsystem
  Through PHY 3.00u : Subsystem
  Through refdes14.0 : Subsystem
